simulink model "ofdm_txrx_mimo_2x"  (Simulink 6.6)
settings: Solver=VariableStepDiscrete  StartTime=0.0  StopTime=20e3  RelTol=1e-3
PreLoadFcn:
  | ofdm_rx_mimo_init
InitFcn:
  | ofdm_rx_mimo_init
StartFcn:
  | ofdm_rx_mimo_init
values from neighbouring files (symbols this model uses):
  BER_RAM_init_size = 2048    [ofdm_tx_mimo_init.m]
  CPLength = 16    [ofdm_tx_mimo_init.m]
  CRCPolynomial = hex2dec('04c11db7')    [ofdm_tx_mimo_init.m]
  freqOffset_track_filtCoef_i = hex2dec('2500')/2^32    [ofdm_rx_mimo_init.m]
  freqOffset_track_filtCoef_p = hex2dec('d0000')/2^32    [ofdm_rx_mimo_init.m]
  hi = zeros(1,length(cci))    [ofdm_rx_mimo_init.m]
  hr = zeros(1,length(ccr))    [ofdm_rx_mimo_init.m]
  max_CP_length = 16    [ofdm_tx_mimo_init.m]
  max_num_baseRateSymbols = 31    [ofdm_tx_mimo_init.m]
  max_num_subcarriers = 64    [ofdm_tx_mimo_init.m]
  max_num_trainingSymbols = 15    [ofdm_tx_mimo_init.m]
  max_numBytes = 16384    [ofdm_tx_mimo_init.m]
  max_OFDM_symbols = 2047    [ofdm_tx_mimo_init.m]
  modConstellation_qam16 = .75*[1 3 -1 -3]./3    [ofdm_tx_mimo_init.m]
  modConstellation_qam256 = 0.9375*[3 1 5 7 11 9 13 15 -3 -1 -5 -7 -11 -9 -13 -15]./15    [ofdm_tx_mimo_init.m]
  modConstellation_qam64 = 0.875*[3 1 5 7 -3 -1 -5 -7]./7    [ofdm_tx_mimo_init.m]
  modConstellation_qpsk = [1 -1]./sqrt(2)    [ofdm_tx_mimo_init.m]
  numBaseRateSymbols = 2    [ofdm_tx_mimo_init.m]
  numSubcarriers = 64    [ofdm_tx_mimo_init.m]
  numTrainingSymbols = 2    [ofdm_tx_mimo_init.m]
  pilot1_indicies = 7 + ( (64-7) * 2^16)    (= 3735559)    [ofdm_tx_mimo_init.m]
  pilot2_indicies = 21 + ( (64-21) * 2^16)    (= 2818069)    [ofdm_tx_mimo_init.m]
  pilotValue_neg = hex2dec('8000') + (2^16 * 0)    [ofdm_tx_mimo_init.m]
  pilotValue_pos = hex2dec('7FFF') + (2^16 * 0)    [ofdm_tx_mimo_init.m]
  pkt_crossCorr_thresh = 0.7    [ofdm_rx_mimo_init.m]
  pkt_energy_thresh = 0    [ofdm_rx_mimo_init.m]
  pkt_numPayloadBytes = 1400+42-6    (= 1436)    [ofdm_tx_mimo_init.m]
  pktByteNums = numHeaderBytes +  				byteIndex_numPayloadBytes(1)*2^8 +  				byteIndex_numPayloadBytes(2)*2^16 +  				byteIndex_simpleDynModMasks * 2^24    [ofdm_rx_mimo_init.m]
  PktDet_Delay = 26 + Symbol_Timing_Offset*2^7    (= 1050)    [ofdm_rx_mimo_init.m]
  preamble = 6*[0 shortsyms_10 longsyms_2]    [ofdm_tx_mimo_init.m]
  preamble_I = real(preamble)    [ofdm_tx_mimo_init.m]
  preamble_Q = imag(preamble)    [ofdm_tx_mimo_init.m]
  RAM_init_size = max_numBytes    (= 16384)    [ofdm_tx_mimo_init.m]
  reg_Interrupt_PktBuf_Control = reg_InterruptControl +  	1 * 2^16 +  	0 * 2^24    (= 65656)    [ofdm_rx_mimo_init.m]
  rx_controlBits = 1 * 2  		 + 1 * 4  		 + 0 * 8  		 + 1 * 16 * rx_SISO_Mode  		 + 1 * 32  		 + 1 * 64  		 + 0 * 128  		 + 1 * 256  		 + 0 * 512  		 + 1 * 1024  		 + 0 * 2048  		 + 1 * 4096  		 + 1 * 8192  		 + 0 * 16384  		 + 1 * 65536  		 + 1 * 131072  		 + 0 * 262144  		 + 1 * 524288    [ofdm_rx_mimo_init.m]
  Rx_PhaseNoiseTrack_K = 0.6094    [ofdm_rx_mimo_init.m]
  rx_postEq_scaling = round(rxScaling*2^11) * (1 + 2^16)    [ofdm_rx_mimo_init.m]
  RxDataScrambling_Seq = [40 198 78 63 82 173 102 245 48 111 172 115 147 230 216 93 72 65 62 2 205 242 122 90 128 83 105 97 73 10 5 252]    [ofdm_tx_mimo_init.m]
  simOnly_numSamples = length(preamble)+( (numSubcarriers+CPLength)*(numTrainingSymbols + numBaseRateSymbols + numFullRateSymbols/2) )    [ofdm_tx_mimo_init.m]
  train = [train train]    [ofdm_tx_mimo_init.m]
  tx_controlBits = (antB_preambleShift * 2^4) + (2^0 * tx_SISO_Mode) + 4*0 + 8*1    (= 73)    [ofdm_tx_mimo_init.m]
  TxDataScrambling_Seq = [40 198 78 63 82 173 102 245 48 111 172 115 147 230 216 93 72 65 62 2 205 242 122 90 128 83 105 97 73 10 5 252]    [ofdm_tx_mimo_init.m]
  txReg_symbolCounts = (2^16 * numFullRateSymbols) + (2^8 *numBaseRateSymbols ) + numTrainingSymbols    [ofdm_tx_mimo_init.m]
  TxRx_FFTScaling = bin2dec('010111') + (bin2dec('000101') * 2^6)    [ofdm_tx_mimo_init.m]
undefined symbols (not defined in the file or in its folder): AntA, AntB, BaseRate, CRC_table_gen, gcbh, intfilt, pktTiming_count_payloadStart, pktTiming_symbol_length, xlBlockLoadCallback, xlGetSimulinkPeriod
libraries: xbsIndex_r4 x3973, dspsigops x4, commchan3 x2, commrflib2 x2, dspsrcs4 x1
(part 11 of 38)

== system ofdm_txrx_mimo_2x/OFDM Rx MIMO/Long Corr 4x/C4 ==
  x : Inport
  AddSub : xbsIndex_r4/AddSub  Orientation=down  mode=Addition  use_carryin=off  use_carryout=off  en=off  latency=0  precision=Full  arith_type=Unsigned  n_bits=8  bin_pt=2  quantization=Truncate  overflow=Wrap  dbl_ovrd=off  use_behavioral_HDL=on  pipelined=off  use_rpm=off  hw_selection=Fabric  block_type=addsub
      settings: xl_area=[3 0 0 4 0 0 0]  block_version=VER_STRING_GOES_HERE
  AddSub{1..7,9..14} (13 alike) : xbsIndex_r4/AddSub  Orientation=down  mode=Addition  use_carryin=off  use_carryout=off  en=off  latency=1  precision=Full  arith_type=Unsigned  n_bits=8  bin_pt=2  quantization=Truncate  overflow=Wrap  dbl_ovrd=off  use_behavioral_HDL=on  pipelined=off  use_rpm=off  hw_selection=Fabric  block_type=addsub
      AddSub1 settings: xl_area=[3 5 0 4 0 0 0]  block_version=VER_STRING_GOES_HERE
      AddSub10 settings: xl_area=[3 5 0 4 0 0 0]  block_version=VER_STRING_GOES_HERE
      AddSub11 settings: xl_area=[3 5 0 4 0 0 0]  block_version=VER_STRING_GOES_HERE
      AddSub12 settings: xl_area=[3 5 0 4 0 0 0]  block_version=VER_STRING_GOES_HERE
      AddSub13 settings: xl_area=[3 6 0 5 0 0 0]  block_version=VER_STRING_GOES_HERE
      AddSub14 settings: xl_area=[3 6 0 5 0 0 0]  block_version=VER_STRING_GOES_HERE
      AddSub2 settings: xl_area=[3 6 0 5 0 0 0]  block_version=VER_STRING_GOES_HERE
      AddSub3 settings: xl_area=[3 5 0 4 0 0 0]  block_version=VER_STRING_GOES_HERE
      AddSub4 settings: xl_area=[4 7 0 6 0 0 0]  block_version=VER_STRING_GOES_HERE
      AddSub5 settings: xl_area=[3 5 0 4 0 0 0]  block_version=VER_STRING_GOES_HERE
      AddSub6 settings: xl_area=[3 6 0 5 0 0 0]  block_version=VER_STRING_GOES_HERE
      AddSub7 settings: xl_area=[3 5 0 4 0 0 0]  block_version=VER_STRING_GOES_HERE
      AddSub9 settings: xl_area=[4 7 0 6 0 0 0]  block_version=VER_STRING_GOES_HERE
  AddSub8 : xbsIndex_r4/AddSub  mode=Addition  use_carryin=off  use_carryout=off  en=off  latency=1  precision=Full  arith_type=Unsigned  n_bits=8  bin_pt=2  quantization=Truncate  overflow=Wrap  dbl_ovrd=off  use_behavioral_HDL=on  pipelined=off  use_rpm=off  hw_selection=Fabric  block_type=addsub
      settings: xl_area=[4 8 0 7 0 0 0]  block_version=VER_STRING_GOES_HERE
  C1 : Subsystem  mask{coef2=h(1:N)}
      mask prompts: coef2 "Coefficients"
  C10 : Subsystem  mask{coef2=h(9*N+1:10*N)}
      mask prompts: coef2 "Coefficients"
  C11 : Subsystem  mask{coef2=h(10*N+1:11*N)}
      mask prompts: coef2 "Coefficients"
  C12 : Subsystem  mask{coef2=h(11*N+1:12*N)}
      mask prompts: coef2 "Coefficients"
  C13 : Subsystem  mask{coef2=h(12*N+1:13*N)}
      mask prompts: coef2 "Coefficients"
  C14 : Subsystem  mask{coef2=h(13*N+1:14*N)}
      mask prompts: coef2 "Coefficients"
  C15 : Subsystem  mask{coef2=h(14*N+1:15*N)}
      mask prompts: coef2 "Coefficients"
  C16 : Subsystem  mask{coef2=h(15*N+1:16*N)}
      mask prompts: coef2 "Coefficients"
  C2 : Subsystem  mask{coef2=h(N+1:2*N)}
      mask prompts: coef2 "Coefficients"
  C3 : Subsystem  mask{coef2=h(2*N+1:3*N)}
      mask prompts: coef2 "Coefficients"
  C4 : Subsystem  mask{coef2=h(3*N+1:4*N)}
      mask prompts: coef2 "Coefficients"
  C5 : Subsystem  mask{coef2=h(4*N+1:5*N)}
      mask prompts: coef2 "Coefficients"
  C6 : Subsystem  mask{coef2=h(5*N+1:6*N)}
      mask prompts: coef2 "Coefficients"
  C7 : Subsystem  mask{coef2=h(6*N+1:7*N)}
      mask prompts: coef2 "Coefficients"
  C8 : Subsystem  mask{coef2=h(7*N+1:8*N)}
      mask prompts: coef2 "Coefficients"
  C9 : Subsystem  mask{coef2=h(8*N+1:9*N)}
      mask prompts: coef2 "Coefficients"
  Delay{1,3..4,6,8,12..13,15} (8 alike) : xbsIndex_r4/Delay  Orientation=down  en=off  latency=N-1  dbl_ovrd=off  reg_retiming=off  block_type=delay
      settings: infoedit=Hardware notes: A delay line is a chain, each link of which is an SRL16 followed by a flip-flop. If register retiming is enabled, the delay line is a chain of flip-flops.  xl_area=[2 4 0 4 0 0 0]  block_version=VER_STRING_GOES_HERE
  Delay10 : xbsIndex_r4/Delay  Orientation=down  en=off  latency=((N-1)*12)-1  dbl_ovrd=off  reg_retiming=off  block_type=delay
      settings: infoedit=Hardware notes: A delay line is a chain, each link of which is an SRL16 followed by a flip-flop. If register retiming is enabled, the delay line is a chain of flip-flops.  xl_area=[9 15 0 15 0 0 0]  block_version=VER_STRING_GOES_HERE
  Delay11 : xbsIndex_r4/Delay  Orientation=down  en=off  latency=((N-1)*14)-1  dbl_ovrd=off  reg_retiming=off  block_type=delay
      settings: infoedit=Hardware notes: A delay line is a chain, each link of which is an SRL16 followed by a flip-flop. If register retiming is enabled, the delay line is a chain of flip-flops.  xl_area=[9 15 0 15 0 0 0]  block_version=VER_STRING_GOES_HERE
  Delay14 : xbsIndex_r4/Delay  Orientation=down  en=off  latency=((N-1)*2)-1  dbl_ovrd=off  reg_retiming=off  block_type=delay
      settings: infoedit=Hardware notes: A delay line is a chain, each link of which is an SRL16 followed by a flip-flop. If register retiming is enabled, the delay line is a chain of flip-flops.  xl_area=[3 5 0 5 0 0 0]  block_version=VER_STRING_GOES_HERE
  Delay2 : xbsIndex_r4/Delay  Orientation=down  en=off  latency=((N-1)*4)-1  dbl_ovrd=off  reg_retiming=off  block_type=delay
      settings: infoedit=Hardware notes: A delay line is a chain, each link of which is an SRL16 followed by a flip-flop. If register retiming is enabled, the delay line is a chain of flip-flops.  xl_area=[3 5 0 5 0 0 0]  block_version=VER_STRING_GOES_HERE
  Delay5 : xbsIndex_r4/Delay  Orientation=down  en=off  latency=((N-1)*6)-1  dbl_ovrd=off  reg_retiming=off  block_type=delay
      settings: infoedit=Hardware notes: A delay line is a chain, each link of which is an SRL16 followed by a flip-flop. If register retiming is enabled, the delay line is a chain of flip-flops.  xl_area=[6 10 0 10 0 0 0]  block_version=VER_STRING_GOES_HERE
  Delay7 : xbsIndex_r4/Delay  Orientation=down  en=off  latency=((N-1)*8)-1  dbl_ovrd=off  reg_retiming=off  block_type=delay
      settings: infoedit=Hardware notes: A delay line is a chain, each link of which is an SRL16 followed by a flip-flop. If register retiming is enabled, the delay line is a chain of flip-flops.  xl_area=[6 10 0 10 0 0 0]  block_version=VER_STRING_GOES_HERE
  Delay9 : xbsIndex_r4/Delay  Orientation=down  en=off  latency=((N-1)*10)-1  dbl_ovrd=off  reg_retiming=off  block_type=delay
      settings: infoedit=Hardware notes: A delay line is a chain, each link of which is an SRL16 followed by a flip-flop. If register retiming is enabled, the delay line is a chain of flip-flops.  xl_area=[9 15 0 15 0 0 0]  block_version=VER_STRING_GOES_HERE
  FSM : Subsystem
  Gateway Out6 : xbsIndex_r4/Gateway Out  hdl_port=off  timing_constraint=None  locs_specified=off  LOCs={}  block_type=gatewayout
      settings: infoedit="Gateway out block.  Converts Xilinx fixed point inputs into ouputs of type Simulink integer, double, or fixed point.<P><P>Hardware notes:  In hardware these blocks become top level output ports or are discarded, depending on how they are configured."  xl_area=[0 0 0 0 0 0 0]  block_version=VER_STRING_GOES_HERE
  Gateway Out7 : xbsIndex_r4/Gateway Out  hdl_port=off  timing_constraint=None  locs_specified=off  LOCs={}  block_type=gatewayout
      settings: infoedit="Gateway out block.  Converts Xilinx fixed point inputs into ouputs of type Simulink integer, double, or fixed point.<P><P>Hardware notes:  In hardware these blocks become top level output ports or are discarded, depending on how they are configured."  xl_area=[0 0 0 0 0 0 0]  block_version=VER_STRING_GOES_HERE
  Gateway Out8 : xbsIndex_r4/Gateway Out  hdl_port=off  timing_constraint=None  locs_specified=off  LOCs={}  block_type=gatewayout
      settings: infoedit="Gateway out block.  Converts Xilinx fixed point inputs into ouputs of type Simulink integer, double, or fixed point.<P><P>Hardware notes:  In hardware these blocks become top level output ports or are discarded, depending on how they are configured."  xl_area=[0 0 0 0 0 0 0]  block_version=VER_STRING_GOES_HERE
  Long Corr Scope2 : Scope  NumInputPorts=3
      settings: YMin=-0.35~-0.175~0  YMax=0.35~0.225~1300  SaveName=ScopeData8
  y : Outport
  wires:
  AddSub9/1 -> Gateway Out8/1, AddSub8/2
  AddSub6/1 -> AddSub9/1, Gateway Out7/1
  C13/1(yn) -> AddSub10/1
  AddSub8/1 -> y/1
  Delay9/1 -> AddSub6/2
  Delay7/1 -> AddSub6/1
  C12/1(yn) -> Delay8/1
  AddSub7/1 -> Delay9/1
  Delay8/1 -> AddSub7/2
  C11/1(yn) -> AddSub7/1
  AddSub4/1 -> AddSub8/1
  AddSub2/1 -> AddSub4/2
  AddSub13/1 -> AddSub4/1
  Delay5/1 -> AddSub2/2
  Delay2/1 -> AddSub2/1
  AddSub/1 -> AddSub13/1
  Delay14/1 -> AddSub13/2
  C8/2(xnz) -> [xnz]
  C10/1(yn) -> Delay6/1
  AddSub5/1 -> Delay7/1
  Delay6/1 -> AddSub5/2
  C9/1(yn) -> AddSub5/1
  C8/1(yn) -> Delay4/1
  C7/1(yn) -> AddSub3/1
  AddSub3/1 -> Delay5/1
  Delay4/1 -> AddSub3/2
  C7/2(xnz) -> C8/1(xn)
  C6/1(yn) -> Delay1/1
  AddSub1/1 -> Delay2/1
  Delay1/1 -> AddSub1/2
  C5/1(yn) -> AddSub1/1
  C1/1(yn) -> AddSub/1
  Delay3/1 -> AddSub/2
  AddSub12/1 -> Delay14/1
  Delay13/1 -> AddSub12/2
  C12/2(xnz) -> C13/1(xn)
  C11/2(xnz) -> C12/1(xn)
  C10/2(xnz) -> C11/1(xn)
  C9/2(xnz) -> C10/1(xn)
  [xnz] -> C9/1(xn)
  C6/2(xnz) -> C7/1(xn)
  C5/2(xnz) -> C6/1(xn)
  C4/1(yn) -> Delay13/1
  C4/2(xnz) -> C5/1(xn)
  C3/1(yn) -> AddSub12/1
  C3/2(xnz) -> C4/1(xn)
  C2/2(xnz) -> C3/1(xn)
  FSM/3(load) -> C12/4(LD), C13/4(LD), C14/4(LD), C16/4(LD), C15/4(LD), C11/4(LD), C10/4(LD), C9/4(LD), C1/4(LD), C8/4(LD), C7/4(LD), C6/4(LD), C5/4(LD), C4/4(LD), C3/4(LD), C2/4(LD)
  FSM/2(Coef Addr) -> C12/3(CAddr), C13/3(CAddr), C14/3(CAddr), C16/3(CAddr), C15/3(CAddr), C11/3(CAddr), C10/3(CAddr), C9/3(CAddr), C1/3(CAddr), C8/3(CAddr), C7/3(CAddr), C6/3(CAddr), C5/3(CAddr), C4/3(CAddr), C3/3(CAddr), C2/3(CAddr)
  FSM/1(Data Addr) -> C12/2(DAddr), C13/2(DAddr), C14/2(DAddr), C16/2(DAddr), C15/2(DAddr), C11/2(DAddr), C10/2(DAddr), C9/2(DAddr), C1/2(DAddr), C8/2(DAddr), C7/2(DAddr), C6/2(DAddr), C5/2(DAddr), C4/2(DAddr), C3/2(DAddr), C2/2(DAddr)
  C1/2(xnz) -> C2/1(xn)
  C2/1(yn) -> Delay3/1
  x/1 -> C1/1(xn)
  C13/2(xnz) -> C14/1(xn)
  C14/2(xnz) -> C15/1(xn)
  C15/2(xnz) -> C16/1(xn)
  C15/1(yn) -> AddSub11/1
  C14/1(yn) -> Delay12/1
  C16/1(yn) -> Delay15/1
  Delay12/1 -> AddSub10/2
  Delay15/1 -> AddSub11/2
  AddSub10/1 -> Delay10/1
  AddSub11/1 -> Delay11/1
  Delay10/1 -> AddSub14/1
  Delay11/1 -> AddSub14/2
  AddSub14/1 -> AddSub9/2, Gateway Out6/1
  Gateway Out6/1 -> Long Corr Scope2/1
  Gateway Out7/1 -> Long Corr Scope2/2
  Gateway Out8/1 -> Long Corr Scope2/3

== system ofdm_txrx_mimo_2x/OFDM Rx MIMO/Long Corr 4x/C6 ==
  x : Inport
  AddSub : xbsIndex_r4/AddSub  Orientation=down  mode=Addition  use_carryin=off  use_carryout=off  en=off  latency=0  precision=Full  arith_type=Unsigned  n_bits=8  bin_pt=2  quantization=Truncate  overflow=Wrap  dbl_ovrd=off  use_behavioral_HDL=on  pipelined=off  use_rpm=off  hw_selection=Fabric  block_type=addsub
      settings: xl_area=[3 0 0 4 0 0 0]  block_version=VER_STRING_GOES_HERE
  AddSub{1..7,9..14} (13 alike) : xbsIndex_r4/AddSub  Orientation=down  mode=Addition  use_carryin=off  use_carryout=off  en=off  latency=1  precision=Full  arith_type=Unsigned  n_bits=8  bin_pt=2  quantization=Truncate  overflow=Wrap  dbl_ovrd=off  use_behavioral_HDL=on  pipelined=off  use_rpm=off  hw_selection=Fabric  block_type=addsub
      AddSub1 settings: xl_area=[3 5 0 4 0 0 0]  block_version=VER_STRING_GOES_HERE
      AddSub10 settings: xl_area=[3 5 0 4 0 0 0]  block_version=VER_STRING_GOES_HERE
      AddSub11 settings: xl_area=[3 5 0 4 0 0 0]  block_version=VER_STRING_GOES_HERE
      AddSub12 settings: xl_area=[3 5 0 4 0 0 0]  block_version=VER_STRING_GOES_HERE
      AddSub13 settings: xl_area=[3 6 0 5 0 0 0]  block_version=VER_STRING_GOES_HERE
      AddSub14 settings: xl_area=[3 6 0 5 0 0 0]  block_version=VER_STRING_GOES_HERE
      AddSub2 settings: xl_area=[3 6 0 5 0 0 0]  block_version=VER_STRING_GOES_HERE
      AddSub3 settings: xl_area=[3 5 0 4 0 0 0]  block_version=VER_STRING_GOES_HERE
      AddSub4 settings: xl_area=[4 7 0 6 0 0 0]  block_version=VER_STRING_GOES_HERE
      AddSub5 settings: xl_area=[3 5 0 4 0 0 0]  block_version=VER_STRING_GOES_HERE
      AddSub6 settings: xl_area=[3 6 0 5 0 0 0]  block_version=VER_STRING_GOES_HERE
      AddSub7 settings: xl_area=[3 5 0 4 0 0 0]  block_version=VER_STRING_GOES_HERE
      AddSub9 settings: xl_area=[4 7 0 6 0 0 0]  block_version=VER_STRING_GOES_HERE
  AddSub8 : xbsIndex_r4/AddSub  mode=Addition  use_carryin=off  use_carryout=off  en=off  latency=1  precision=Full  arith_type=Unsigned  n_bits=8  bin_pt=2  quantization=Truncate  overflow=Wrap  dbl_ovrd=off  use_behavioral_HDL=on  pipelined=off  use_rpm=off  hw_selection=Fabric  block_type=addsub
      settings: xl_area=[4 8 0 7 0 0 0]  block_version=VER_STRING_GOES_HERE
  C1 : Subsystem  mask{coef2=h(1:N)}
      mask prompts: coef2 "Coefficients"
  C10 : Subsystem  mask{coef2=h(9*N+1:10*N)}
      mask prompts: coef2 "Coefficients"
  C11 : Subsystem  mask{coef2=h(10*N+1:11*N)}
      mask prompts: coef2 "Coefficients"
  C12 : Subsystem  mask{coef2=h(11*N+1:12*N)}
      mask prompts: coef2 "Coefficients"
  C13 : Subsystem  mask{coef2=h(12*N+1:13*N)}
      mask prompts: coef2 "Coefficients"
  C14 : Subsystem  mask{coef2=h(13*N+1:14*N)}
      mask prompts: coef2 "Coefficients"
  C15 : Subsystem  mask{coef2=h(14*N+1:15*N)}
      mask prompts: coef2 "Coefficients"
  C16 : Subsystem  mask{coef2=h(15*N+1:16*N)}
      mask prompts: coef2 "Coefficients"
  C2 : Subsystem  mask{coef2=h(N+1:2*N)}
      mask prompts: coef2 "Coefficients"
  C3 : Subsystem  mask{coef2=h(2*N+1:3*N)}
      mask prompts: coef2 "Coefficients"
  C4 : Subsystem  mask{coef2=h(3*N+1:4*N)}
      mask prompts: coef2 "Coefficients"
  C5 : Subsystem  mask{coef2=h(4*N+1:5*N)}
      mask prompts: coef2 "Coefficients"
  C6 : Subsystem  mask{coef2=h(5*N+1:6*N)}
      mask prompts: coef2 "Coefficients"
  C7 : Subsystem  mask{coef2=h(6*N+1:7*N)}
      mask prompts: coef2 "Coefficients"
  C8 : Subsystem  mask{coef2=h(7*N+1:8*N)}
      mask prompts: coef2 "Coefficients"
  C9 : Subsystem  mask{coef2=h(8*N+1:9*N)}
      mask prompts: coef2 "Coefficients"
  Delay{1,3..4,6,8,12..13,15} (8 alike) : xbsIndex_r4/Delay  Orientation=down  en=off  latency=N-1  dbl_ovrd=off  reg_retiming=off  block_type=delay
      settings: infoedit=Hardware notes: A delay line is a chain, each link of which is an SRL16 followed by a flip-flop. If register retiming is enabled, the delay line is a chain of flip-flops.  xl_area=[2 4 0 4 0 0 0]  block_version=VER_STRING_GOES_HERE
  Delay10 : xbsIndex_r4/Delay  Orientation=down  en=off  latency=((N-1)*12)-1  dbl_ovrd=off  reg_retiming=off  block_type=delay
      settings: infoedit=Hardware notes: A delay line is a chain, each link of which is an SRL16 followed by a flip-flop. If register retiming is enabled, the delay line is a chain of flip-flops.  xl_area=[9 15 0 15 0 0 0]  block_version=VER_STRING_GOES_HERE
  Delay11 : xbsIndex_r4/Delay  Orientation=down  en=off  latency=((N-1)*14)-1  dbl_ovrd=off  reg_retiming=off  block_type=delay
      settings: infoedit=Hardware notes: A delay line is a chain, each link of which is an SRL16 followed by a flip-flop. If register retiming is enabled, the delay line is a chain of flip-flops.  xl_area=[9 15 0 15 0 0 0]  block_version=VER_STRING_GOES_HERE
  Delay14 : xbsIndex_r4/Delay  Orientation=down  en=off  latency=((N-1)*2)-1  dbl_ovrd=off  reg_retiming=off  block_type=delay
      settings: infoedit=Hardware notes: A delay line is a chain, each link of which is an SRL16 followed by a flip-flop. If register retiming is enabled, the delay line is a chain of flip-flops.  xl_area=[3 5 0 5 0 0 0]  block_version=VER_STRING_GOES_HERE
  Delay2 : xbsIndex_r4/Delay  Orientation=down  en=off  latency=((N-1)*4)-1  dbl_ovrd=off  reg_retiming=off  block_type=delay
      settings: infoedit=Hardware notes: A delay line is a chain, each link of which is an SRL16 followed by a flip-flop. If register retiming is enabled, the delay line is a chain of flip-flops.  xl_area=[3 5 0 5 0 0 0]  block_version=VER_STRING_GOES_HERE
  Delay5 : xbsIndex_r4/Delay  Orientation=down  en=off  latency=((N-1)*6)-1  dbl_ovrd=off  reg_retiming=off  block_type=delay
      settings: infoedit=Hardware notes: A delay line is a chain, each link of which is an SRL16 followed by a flip-flop. If register retiming is enabled, the delay line is a chain of flip-flops.  xl_area=[6 10 0 10 0 0 0]  block_version=VER_STRING_GOES_HERE
  Delay7 : xbsIndex_r4/Delay  Orientation=down  en=off  latency=((N-1)*8)-1  dbl_ovrd=off  reg_retiming=off  block_type=delay
      settings: infoedit=Hardware notes: A delay line is a chain, each link of which is an SRL16 followed by a flip-flop. If register retiming is enabled, the delay line is a chain of flip-flops.  xl_area=[6 10 0 10 0 0 0]  block_version=VER_STRING_GOES_HERE
  Delay9 : xbsIndex_r4/Delay  Orientation=down  en=off  latency=((N-1)*10)-1  dbl_ovrd=off  reg_retiming=off  block_type=delay
      settings: infoedit=Hardware notes: A delay line is a chain, each link of which is an SRL16 followed by a flip-flop. If register retiming is enabled, the delay line is a chain of flip-flops.  xl_area=[9 15 0 15 0 0 0]  block_version=VER_STRING_GOES_HERE
  FSM : Subsystem
  Gateway Out6 : xbsIndex_r4/Gateway Out  hdl_port=off  timing_constraint=None  locs_specified=off  LOCs={}  block_type=gatewayout
      settings: infoedit="Gateway out block.  Converts Xilinx fixed point inputs into ouputs of type Simulink integer, double, or fixed point.<P><P>Hardware notes:  In hardware these blocks become top level output ports or are discarded, depending on how they are configured."  xl_area=[0 0 0 0 0 0 0]  block_version=VER_STRING_GOES_HERE
  Gateway Out7 : xbsIndex_r4/Gateway Out  hdl_port=off  timing_constraint=None  locs_specified=off  LOCs={}  block_type=gatewayout
      settings: infoedit="Gateway out block.  Converts Xilinx fixed point inputs into ouputs of type Simulink integer, double, or fixed point.<P><P>Hardware notes:  In hardware these blocks become top level output ports or are discarded, depending on how they are configured."  xl_area=[0 0 0 0 0 0 0]  block_version=VER_STRING_GOES_HERE
  Gateway Out8 : xbsIndex_r4/Gateway Out  hdl_port=off  timing_constraint=None  locs_specified=off  LOCs={}  block_type=gatewayout
      settings: infoedit="Gateway out block.  Converts Xilinx fixed point inputs into ouputs of type Simulink integer, double, or fixed point.<P><P>Hardware notes:  In hardware these blocks become top level output ports or are discarded, depending on how they are configured."  xl_area=[0 0 0 0 0 0 0]  block_version=VER_STRING_GOES_HERE
  Long Corr Scope2 : Scope  NumInputPorts=3
      settings: YMin=-0.35~-0.175~0  YMax=0.35~0.225~1300  SaveName=ScopeData8
  y : Outport
  wires:
  Gateway Out8/1 -> Long Corr Scope2/3
  Gateway Out7/1 -> Long Corr Scope2/2
  Gateway Out6/1 -> Long Corr Scope2/1
  AddSub14/1 -> Gateway Out6/1, AddSub9/2
  Delay11/1 -> AddSub14/2
  Delay10/1 -> AddSub14/1
  AddSub11/1 -> Delay11/1
  AddSub10/1 -> Delay10/1
  Delay15/1 -> AddSub11/2
  Delay12/1 -> AddSub10/2
  C16/1(yn) -> Delay15/1
  C14/1(yn) -> Delay12/1
  C15/1(yn) -> AddSub11/1
  C15/2(xnz) -> C16/1(xn)
  C14/2(xnz) -> C15/1(xn)
  C13/2(xnz) -> C14/1(xn)
  x/1 -> C1/1(xn)
  C2/1(yn) -> Delay3/1
  C1/2(xnz) -> C2/1(xn)
  FSM/1(Data Addr) -> C2/2(DAddr), C3/2(DAddr), C4/2(DAddr), C5/2(DAddr), C6/2(DAddr), C7/2(DAddr), C8/2(DAddr), C1/2(DAddr), C9/2(DAddr), C10/2(DAddr), C11/2(DAddr), C15/2(DAddr), C16/2(DAddr), C14/2(DAddr), C13/2(DAddr), C12/2(DAddr)
  FSM/2(Coef Addr) -> C2/3(CAddr), C3/3(CAddr), C4/3(CAddr), C5/3(CAddr), C6/3(CAddr), C7/3(CAddr), C8/3(CAddr), C1/3(CAddr), C9/3(CAddr), C10/3(CAddr), C11/3(CAddr), C15/3(CAddr), C16/3(CAddr), C14/3(CAddr), C13/3(CAddr), C12/3(CAddr)
  FSM/3(load) -> C2/4(LD), C3/4(LD), C4/4(LD), C5/4(LD), C6/4(LD), C7/4(LD), C8/4(LD), C1/4(LD), C9/4(LD), C10/4(LD), C11/4(LD), C15/4(LD), C16/4(LD), C14/4(LD), C13/4(LD), C12/4(LD)
  C2/2(xnz) -> C3/1(xn)
  C3/2(xnz) -> C4/1(xn)
  C3/1(yn) -> AddSub12/1
  C4/2(xnz) -> C5/1(xn)
  C4/1(yn) -> Delay13/1
  C5/2(xnz) -> C6/1(xn)
  C6/2(xnz) -> C7/1(xn)
  [xnz] -> C9/1(xn)
  C9/2(xnz) -> C10/1(xn)
  C10/2(xnz) -> C11/1(xn)
  C11/2(xnz) -> C12/1(xn)
  C12/2(xnz) -> C13/1(xn)
  Delay13/1 -> AddSub12/2
  AddSub12/1 -> Delay14/1
  Delay3/1 -> AddSub/2
  C1/1(yn) -> AddSub/1
  C5/1(yn) -> AddSub1/1
  Delay1/1 -> AddSub1/2
  AddSub1/1 -> Delay2/1
  C6/1(yn) -> Delay1/1
  C7/2(xnz) -> C8/1(xn)
  Delay4/1 -> AddSub3/2
  AddSub3/1 -> Delay5/1
  C7/1(yn) -> AddSub3/1
  C8/1(yn) -> Delay4/1
  C9/1(yn) -> AddSub5/1
  Delay6/1 -> AddSub5/2
  AddSub5/1 -> Delay7/1
  C10/1(yn) -> Delay6/1
  C8/2(xnz) -> [xnz]
  Delay14/1 -> AddSub13/2
  AddSub/1 -> AddSub13/1
  Delay2/1 -> AddSub2/1
  Delay5/1 -> AddSub2/2
  AddSub13/1 -> AddSub4/1
  AddSub2/1 -> AddSub4/2
  AddSub4/1 -> AddSub8/1
  C11/1(yn) -> AddSub7/1
  Delay8/1 -> AddSub7/2
  AddSub7/1 -> Delay9/1
  C12/1(yn) -> Delay8/1
  Delay7/1 -> AddSub6/1
  Delay9/1 -> AddSub6/2
  AddSub8/1 -> y/1
  C13/1(yn) -> AddSub10/1
  AddSub6/1 -> Gateway Out7/1, AddSub9/1
  AddSub9/1 -> AddSub8/2, Gateway Out8/1

== system ofdm_txrx_mimo_2x/OFDM Rx MIMO/Long Corr 4x/C7 ==
  x : Inport
  AddSub : xbsIndex_r4/AddSub  Orientation=down  mode=Addition  use_carryin=off  use_carryout=off  en=off  latency=0  precision=Full  arith_type=Unsigned  n_bits=8  bin_pt=2  quantization=Truncate  overflow=Wrap  dbl_ovrd=off  use_behavioral_HDL=on  pipelined=off  use_rpm=off  hw_selection=Fabric  block_type=addsub
      settings: xl_area=[3 0 0 4 0 0 0]  block_version=VER_STRING_GOES_HERE
  AddSub{1..7,9..14} (13 alike) : xbsIndex_r4/AddSub  Orientation=down  mode=Addition  use_carryin=off  use_carryout=off  en=off  latency=1  precision=Full  arith_type=Unsigned  n_bits=8  bin_pt=2  quantization=Truncate  overflow=Wrap  dbl_ovrd=off  use_behavioral_HDL=on  pipelined=off  use_rpm=off  hw_selection=Fabric  block_type=addsub
      AddSub1 settings: xl_area=[3 5 0 4 0 0 0]  block_version=VER_STRING_GOES_HERE
      AddSub10 settings: xl_area=[3 5 0 4 0 0 0]  block_version=VER_STRING_GOES_HERE
      AddSub11 settings: xl_area=[3 5 0 4 0 0 0]  block_version=VER_STRING_GOES_HERE
      AddSub12 settings: xl_area=[3 5 0 4 0 0 0]  block_version=VER_STRING_GOES_HERE
      AddSub13 settings: xl_area=[3 6 0 5 0 0 0]  block_version=VER_STRING_GOES_HERE
      AddSub14 settings: xl_area=[3 6 0 5 0 0 0]  block_version=VER_STRING_GOES_HERE
      AddSub2 settings: xl_area=[3 6 0 5 0 0 0]  block_version=VER_STRING_GOES_HERE
      AddSub3 settings: xl_area=[3 5 0 4 0 0 0]  block_version=VER_STRING_GOES_HERE
      AddSub4 settings: xl_area=[4 7 0 6 0 0 0]  block_version=VER_STRING_GOES_HERE
      AddSub5 settings: xl_area=[3 5 0 4 0 0 0]  block_version=VER_STRING_GOES_HERE
      AddSub6 settings: xl_area=[3 6 0 5 0 0 0]  block_version=VER_STRING_GOES_HERE
      AddSub7 settings: xl_area=[3 5 0 4 0 0 0]  block_version=VER_STRING_GOES_HERE
      AddSub9 settings: xl_area=[4 7 0 6 0 0 0]  block_version=VER_STRING_GOES_HERE
  AddSub8 : xbsIndex_r4/AddSub  mode=Addition  use_carryin=off  use_carryout=off  en=off  latency=1  precision=Full  arith_type=Unsigned  n_bits=8  bin_pt=2  quantization=Truncate  overflow=Wrap  dbl_ovrd=off  use_behavioral_HDL=on  pipelined=off  use_rpm=off  hw_selection=Fabric  block_type=addsub
      settings: xl_area=[4 8 0 7 0 0 0]  block_version=VER_STRING_GOES_HERE
  C1 : Subsystem  mask{coef2=h(1:N)}
      mask prompts: coef2 "Coefficients"
  C10 : Subsystem  mask{coef2=h(9*N+1:10*N)}
      mask prompts: coef2 "Coefficients"
  C11 : Subsystem  mask{coef2=h(10*N+1:11*N)}
      mask prompts: coef2 "Coefficients"
  C12 : Subsystem  mask{coef2=h(11*N+1:12*N)}
      mask prompts: coef2 "Coefficients"
  C13 : Subsystem  mask{coef2=h(12*N+1:13*N)}
      mask prompts: coef2 "Coefficients"
  C14 : Subsystem  mask{coef2=h(13*N+1:14*N)}
      mask prompts: coef2 "Coefficients"
  C15 : Subsystem  mask{coef2=h(14*N+1:15*N)}
      mask prompts: coef2 "Coefficients"
  C16 : Subsystem  mask{coef2=h(15*N+1:16*N)}
      mask prompts: coef2 "Coefficients"
  C2 : Subsystem  mask{coef2=h(N+1:2*N)}
      mask prompts: coef2 "Coefficients"
  C3 : Subsystem  mask{coef2=h(2*N+1:3*N)}
      mask prompts: coef2 "Coefficients"
  C4 : Subsystem  mask{coef2=h(3*N+1:4*N)}
      mask prompts: coef2 "Coefficients"
  C5 : Subsystem  mask{coef2=h(4*N+1:5*N)}
      mask prompts: coef2 "Coefficients"
  C6 : Subsystem  mask{coef2=h(5*N+1:6*N)}
      mask prompts: coef2 "Coefficients"
  C7 : Subsystem  mask{coef2=h(6*N+1:7*N)}
      mask prompts: coef2 "Coefficients"
  C8 : Subsystem  mask{coef2=h(7*N+1:8*N)}
      mask prompts: coef2 "Coefficients"
  C9 : Subsystem  mask{coef2=h(8*N+1:9*N)}
      mask prompts: coef2 "Coefficients"
  Delay{1,3..4,6,8,12..13,15} (8 alike) : xbsIndex_r4/Delay  Orientation=down  en=off  latency=N-1  dbl_ovrd=off  reg_retiming=off  block_type=delay
      settings: infoedit=Hardware notes: A delay line is a chain, each link of which is an SRL16 followed by a flip-flop. If register retiming is enabled, the delay line is a chain of flip-flops.  xl_area=[2 4 0 4 0 0 0]  block_version=VER_STRING_GOES_HERE
  Delay10 : xbsIndex_r4/Delay  Orientation=down  en=off  latency=((N-1)*12)-1  dbl_ovrd=off  reg_retiming=off  block_type=delay
      settings: infoedit=Hardware notes: A delay line is a chain, each link of which is an SRL16 followed by a flip-flop. If register retiming is enabled, the delay line is a chain of flip-flops.  xl_area=[9 15 0 15 0 0 0]  block_version=VER_STRING_GOES_HERE
  Delay11 : xbsIndex_r4/Delay  Orientation=down  en=off  latency=((N-1)*14)-1  dbl_ovrd=off  reg_retiming=off  block_type=delay
      settings: infoedit=Hardware notes: A delay line is a chain, each link of which is an SRL16 followed by a flip-flop. If register retiming is enabled, the delay line is a chain of flip-flops.  xl_area=[9 15 0 15 0 0 0]  block_version=VER_STRING_GOES_HERE
  Delay14 : xbsIndex_r4/Delay  Orientation=down  en=off  latency=((N-1)*2)-1  dbl_ovrd=off  reg_retiming=off  block_type=delay
      settings: infoedit=Hardware notes: A delay line is a chain, each link of which is an SRL16 followed by a flip-flop. If register retiming is enabled, the delay line is a chain of flip-flops.  xl_area=[3 5 0 5 0 0 0]  block_version=VER_STRING_GOES_HERE
  Delay2 : xbsIndex_r4/Delay  Orientation=down  en=off  latency=((N-1)*4)-1  dbl_ovrd=off  reg_retiming=off  block_type=delay
      settings: infoedit=Hardware notes: A delay line is a chain, each link of which is an SRL16 followed by a flip-flop. If register retiming is enabled, the delay line is a chain of flip-flops.  xl_area=[3 5 0 5 0 0 0]  block_version=VER_STRING_GOES_HERE
  Delay5 : xbsIndex_r4/Delay  Orientation=down  en=off  latency=((N-1)*6)-1  dbl_ovrd=off  reg_retiming=off  block_type=delay
      settings: infoedit=Hardware notes: A delay line is a chain, each link of which is an SRL16 followed by a flip-flop. If register retiming is enabled, the delay line is a chain of flip-flops.  xl_area=[6 10 0 10 0 0 0]  block_version=VER_STRING_GOES_HERE
  Delay7 : xbsIndex_r4/Delay  Orientation=down  en=off  latency=((N-1)*8)-1  dbl_ovrd=off  reg_retiming=off  block_type=delay
      settings: infoedit=Hardware notes: A delay line is a chain, each link of which is an SRL16 followed by a flip-flop. If register retiming is enabled, the delay line is a chain of flip-flops.  xl_area=[6 10 0 10 0 0 0]  block_version=VER_STRING_GOES_HERE
  Delay9 : xbsIndex_r4/Delay  Orientation=down  en=off  latency=((N-1)*10)-1  dbl_ovrd=off  reg_retiming=off  block_type=delay
      settings: infoedit=Hardware notes: A delay line is a chain, each link of which is an SRL16 followed by a flip-flop. If register retiming is enabled, the delay line is a chain of flip-flops.  xl_area=[9 15 0 15 0 0 0]  block_version=VER_STRING_GOES_HERE
  FSM : Subsystem
  Gateway Out6 : xbsIndex_r4/Gateway Out  hdl_port=off  timing_constraint=None  locs_specified=off  LOCs={}  block_type=gatewayout
      settings: infoedit="Gateway out block.  Converts Xilinx fixed point inputs into ouputs of type Simulink integer, double, or fixed point.<P><P>Hardware notes:  In hardware these blocks become top level output ports or are discarded, depending on how they are configured."  xl_area=[0 0 0 0 0 0 0]  block_version=VER_STRING_GOES_HERE
  Gateway Out7 : xbsIndex_r4/Gateway Out  hdl_port=off  timing_constraint=None  locs_specified=off  LOCs={}  block_type=gatewayout
      settings: infoedit="Gateway out block.  Converts Xilinx fixed point inputs into ouputs of type Simulink integer, double, or fixed point.<P><P>Hardware notes:  In hardware these blocks become top level output ports or are discarded, depending on how they are configured."  xl_area=[0 0 0 0 0 0 0]  block_version=VER_STRING_GOES_HERE
  Gateway Out8 : xbsIndex_r4/Gateway Out  hdl_port=off  timing_constraint=None  locs_specified=off  LOCs={}  block_type=gatewayout
      settings: infoedit="Gateway out block.  Converts Xilinx fixed point inputs into ouputs of type Simulink integer, double, or fixed point.<P><P>Hardware notes:  In hardware these blocks become top level output ports or are discarded, depending on how they are configured."  xl_area=[0 0 0 0 0 0 0]  block_version=VER_STRING_GOES_HERE
  Long Corr Scope2 : Scope  NumInputPorts=3
      settings: YMin=-0.35~-0.175~0  YMax=0.35~0.225~1300  SaveName=ScopeData8
  y : Outport
  wires:
  AddSub9/1 -> Gateway Out8/1, AddSub8/2
  AddSub6/1 -> AddSub9/1, Gateway Out7/1
  C13/1(yn) -> AddSub10/1
  AddSub8/1 -> y/1
  Delay9/1 -> AddSub6/2
  Delay7/1 -> AddSub6/1
  C12/1(yn) -> Delay8/1
  AddSub7/1 -> Delay9/1
  Delay8/1 -> AddSub7/2
  C11/1(yn) -> AddSub7/1
  AddSub4/1 -> AddSub8/1
  AddSub2/1 -> AddSub4/2
  AddSub13/1 -> AddSub4/1
  Delay5/1 -> AddSub2/2
  Delay2/1 -> AddSub2/1
  AddSub/1 -> AddSub13/1
  Delay14/1 -> AddSub13/2
  C8/2(xnz) -> [xnz]
  C10/1(yn) -> Delay6/1
  AddSub5/1 -> Delay7/1
  Delay6/1 -> AddSub5/2
  C9/1(yn) -> AddSub5/1
  C8/1(yn) -> Delay4/1
  C7/1(yn) -> AddSub3/1
  AddSub3/1 -> Delay5/1
  Delay4/1 -> AddSub3/2
  C7/2(xnz) -> C8/1(xn)
  C6/1(yn) -> Delay1/1
  AddSub1/1 -> Delay2/1
  Delay1/1 -> AddSub1/2
  C5/1(yn) -> AddSub1/1
  C1/1(yn) -> AddSub/1
  Delay3/1 -> AddSub/2
  AddSub12/1 -> Delay14/1
  Delay13/1 -> AddSub12/2
  C12/2(xnz) -> C13/1(xn)
  C11/2(xnz) -> C12/1(xn)
  C10/2(xnz) -> C11/1(xn)
  C9/2(xnz) -> C10/1(xn)
  [xnz] -> C9/1(xn)
  C6/2(xnz) -> C7/1(xn)
  C5/2(xnz) -> C6/1(xn)
  C4/1(yn) -> Delay13/1
  C4/2(xnz) -> C5/1(xn)
  C3/1(yn) -> AddSub12/1
  C3/2(xnz) -> C4/1(xn)
  C2/2(xnz) -> C3/1(xn)
  FSM/3(load) -> C12/4(LD), C13/4(LD), C14/4(LD), C16/4(LD), C15/4(LD), C11/4(LD), C10/4(LD), C9/4(LD), C1/4(LD), C8/4(LD), C7/4(LD), C6/4(LD), C5/4(LD), C4/4(LD), C3/4(LD), C2/4(LD)
  FSM/2(Coef Addr) -> C12/3(CAddr), C13/3(CAddr), C14/3(CAddr), C16/3(CAddr), C15/3(CAddr), C11/3(CAddr), C10/3(CAddr), C9/3(CAddr), C1/3(CAddr), C8/3(CAddr), C7/3(CAddr), C6/3(CAddr), C5/3(CAddr), C4/3(CAddr), C3/3(CAddr), C2/3(CAddr)
  FSM/1(Data Addr) -> C12/2(DAddr), C13/2(DAddr), C14/2(DAddr), C16/2(DAddr), C15/2(DAddr), C11/2(DAddr), C10/2(DAddr), C9/2(DAddr), C1/2(DAddr), C8/2(DAddr), C7/2(DAddr), C6/2(DAddr), C5/2(DAddr), C4/2(DAddr), C3/2(DAddr), C2/2(DAddr)
  C1/2(xnz) -> C2/1(xn)
  C2/1(yn) -> Delay3/1
  x/1 -> C1/1(xn)
  C13/2(xnz) -> C14/1(xn)
  C14/2(xnz) -> C15/1(xn)
  C15/2(xnz) -> C16/1(xn)
  C15/1(yn) -> AddSub11/1
  C14/1(yn) -> Delay12/1
  C16/1(yn) -> Delay15/1
  Delay12/1 -> AddSub10/2
  Delay15/1 -> AddSub11/2
  AddSub10/1 -> Delay10/1
  AddSub11/1 -> Delay11/1
  Delay10/1 -> AddSub14/1
  Delay11/1 -> AddSub14/2
  AddSub14/1 -> AddSub9/2, Gateway Out6/1
  Gateway Out6/1 -> Long Corr Scope2/1
  Gateway Out7/1 -> Long Corr Scope2/2
  Gateway Out8/1 -> Long Corr Scope2/3

== system ofdm_txrx_mimo_2x/OFDM Rx MIMO/Packet_Detection/A*conj(B) 4xCLK ==
  Re[A] : Inport
  Re[B] : Inport  Port=2
  Im[A] : Inport  Port=3
  Im[B] : Inport  Port=4
  Add1 : xbsIndex_r4/AddSub  mode=Addition  use_carryin=off  use_carryout=off  en=off  latency=0  precision=User Defined  arith_type="Signed  (2's comp)"  n_bits=17  bin_pt=15  quantization=Truncate  overflow=Flag as error  dbl_ovrd=off  use_behavioral_HDL=on  pipelined=off  use_rpm=on  hw_selection=Fabric  block_type=addsub
      settings: xl_area=[9 0 0 16 0 0 0]  block_version=VER_STRING_GOES_HERE
  Counter : xbsIndex_r4/Counter  Orientation=down  cnt_type=Free Running  cnt_to=4  operation=Up  start_count=0  cnt_by_val=1  arith_type=Unsigned  n_bits=2  bin_pt=0  load_pin=off  rst=off  en=off  explicit_period=on  period=1  dbl_ovrd=off  use_behavioral_HDL=off  use_rpm=on  implementation=Fabric  block_type=counter
      settings: xl_area=[3 3 0 4 0 0 0]  block_version=VER_STRING_GOES_HERE
  Delay1 : xbsIndex_r4/Delay  en=off  latency=1  dbl_ovrd=off  reg_retiming=off  block_type=delay
      settings: infoedit=Hardware notes: A delay line is a chain, each link of which is an SRL16 followed by a flip-flop. If register retiming is enabled, the delay line is a chain of flip-flops.  xl_area=[8 16 0 0 0 0 0]  block_version=VER_STRING_GOES_HERE
  Delay2 : xbsIndex_r4/Delay  en=off  latency=2  dbl_ovrd=off  reg_retiming=off  block_type=delay
      settings: infoedit=Hardware notes: A delay line is a chain, each link of which is an SRL16 followed by a flip-flop. If register retiming is enabled, the delay line is a chain of flip-flops.  xl_area=[9 17 0 17 0 0 0]  block_version=VER_STRING_GOES_HERE
  Delay3 : xbsIndex_r4/Delay  en=off  latency=0  dbl_ovrd=off  reg_retiming=off  block_type=delay
      settings: infoedit=Hardware notes: A delay line is a chain, each link of which is an SRL16 followed by a flip-flop. If register retiming is enabled, the delay line is a chain of flip-flops.  xl_area=[9 17 0 17 0 0 0]  block_version=VER_STRING_GOES_HERE
  Down Sample3 : xbsIndex_r4/Down Sample  sample_ratio=4  sample_phase=First Value of Frame  en=off  latency=1  dbl_ovrd=off  block_type=dsamp
      settings: xl_area=[9 17 0 0 0 0 0]  block_version=VER_STRING_GOES_HERE
  Down Sample4 : xbsIndex_r4/Down Sample  sample_ratio=4  sample_phase=First Value of Frame  en=off  latency=1  dbl_ovrd=off  block_type=dsamp
      settings: xl_area=[9 17 0 0 0 0 0]  block_version=VER_STRING_GOES_HERE
  Mult4 : xbsIndex_r4/Mult  precision=User Defined  arith_type="Signed  (2's comp)"  n_bits=16  bin_pt=15  quantization=Truncate  overflow=Flag as error  en=off  latency=1  dbl_ovrd=off  use_behavioral_HDL=off  use_embedded=on  opt=Speed  optimum_pipeline=off  pipeline=on  use_rpm=off  placement_style=Rectangular shape  block_type=mult
      settings: infoedit=Hardware notes: To use the internal pipeline stage of the dedicated multiplier you must select 'Pipeline for maximum performance'.  xl_area=[14 27 0 27 0 1 0]  block_version=VER_STRING_GOES_HERE
  Mux : xbsIndex_r4/Mux  inputs=4  en=off  latency=0  precision=Full  arith_type="Signed  (2's comp)"  n_bits=16  bin_pt=13  quantization=Truncate  overflow=Wrap  dbl_ovrd=off  block_type=mux
      settings: xl_area=[14 0 0 28 0 0 0]  block_version=VER_STRING_GOES_HERE
  Mux1 : xbsIndex_r4/Mux  inputs=4  en=off  latency=0  precision=Full  arith_type="Signed  (2's comp)"  n_bits=16  bin_pt=13  quantization=Truncate  overflow=Wrap  dbl_ovrd=off  block_type=mux
      settings: xl_area=[14 0 0 28 0 0 0]  block_version=VER_STRING_GOES_HERE
  Negate : xbsIndex_r4/Negate  precision=User Defined  arith_type="Signed  (2's comp)"  n_bits=14  bin_pt=13  quantization=Truncate  overflow=Wrap  en=off  latency=0  dbl_ovrd=off  block_type=negate
      settings: xl_area=[7 0 0 14 0 0 0]  block_version=VER_STRING_GOES_HERE
  Negate1 : xbsIndex_r4/Negate  precision=User Defined  arith_type="Signed  (2's comp)"  n_bits=14  bin_pt=13  quantization=Truncate  overflow=Wrap  en=off  latency=0  dbl_ovrd=off  block_type=negate
      settings: xl_area=[7 0 0 14 0 0 0]  block_version=VER_STRING_GOES_HERE
  Slice : xbsIndex_r4/Slice  nbits=2  boolean_output=off  mode=Lower Bit Location + Width  bit1=0  base1=MSB of Input  bit0=0  base0=LSB of Input  dbl_ovrd=off  block_type=slice
      settings: infoedit="Extracts a given range of bits from each input sample and presents it at the output.  The output type is ordinarily unsigned with binary point at zero, but can be Boolean when the slice is one bit wide.<P><P>Hardware notes: In hardware this block costs nothing."  block_version=VER_STRING_GOES_HERE
  Up Sample{1..4} (4 alike) : xbsIndex_r4/Up Sample  sample_ratio=4  copy_samples=on  dbl_ovrd=off  block_type=usamp
      settings: infoedit="Up samples input data.  Inserted values  can be zeros or copies of the most recent input sample.<P><P>Hardware notes: No hardware is needed if inserted values are copies of the input sample; otherwise, a mux and single bit flip-flop are used."  xl_area=[0 0 0 0 0 0 0]  block_version=VER_STRING_GOES_HERE
  Re : Outport
  Im1 : Outport  Port=2
  wires:
  Delay3/1 -> Down Sample4/1
  Slice/1 -> Mux/1, Mux1/1
  Counter/1 -> Slice/1
  Delay1/1 -> Add1/2
  Mult4/1 -> Delay1/1, Add1/1
  Up Sample1/1 -> Mux1/5, Negate/1
  Up Sample4/1 -> Negate1/1
  Up Sample2/1 -> Mux/2, Mux/5
  Up Sample3/1 -> Mux1/4, Mux1/2
  Re[A]/1 -> Up Sample3/1
  Re[B]/1 -> Up Sample2/1
  Im[A]/1 -> Up Sample1/1
  Im[B]/1 -> Up Sample4/1
  Mux1/1 -> Mult4/1
  Mux/1 -> Mult4/2
  Delay2/1 -> Down Sample3/1
  Down Sample4/1 -> Im1/1
  Down Sample3/1 -> Re/1
  Add1/1 -> Delay2/1, Delay3/1
  Negate1/1 -> Mux/4, Mux/3
  Negate/1 -> Mux1/3

== system ofdm_txrx_mimo_2x/OFDM Rx MIMO/Packet_Detection/Magnitude Squared ==
  I : Inport
  Q : Inport  Port=2
  AddSub1 : xbsIndex_r4/AddSub  mode=Addition  use_carryin=off  use_carryout=off  en=off  latency=addLatency  precision=User Defined  arith_type=Unsigned  n_bits=prec  bin_pt=bp  quantization=Truncate  overflow=Flag as error  dbl_ovrd=off  use_behavioral_HDL=on  pipelined=off  use_rpm=on  hw_selection=Fabric  block_type=addsub
      settings: xl_area=[12 22 0 22 0 0 0]  block_version=VER_STRING_GOES_HERE
  Mult1 : xbsIndex_r4/Mult  precision=User Defined  arith_type="Signed  (2's comp)"  n_bits=prec  bin_pt=bp+1  quantization=Truncate  overflow=Flag as error  en=off  latency=multLatency  dbl_ovrd=off  use_behavioral_HDL=off  use_embedded=on  opt=Speed  optimum_pipeline=off  pipeline=on  use_rpm=off  placement_style=Rectangular shape  block_type=mult
      settings: infoedit=Hardware notes: To use the internal pipeline stage of the dedicated multiplier you must select 'Pipeline for maximum performance'.  xl_area=[63 105 0 106 0 4 0]  block_version=VER_STRING_GOES_HERE
  Mult4 : xbsIndex_r4/Mult  precision=User Defined  arith_type="Signed  (2's comp)"  n_bits=prec  bin_pt=bp+1  quantization=Truncate  overflow=Flag as error  en=off  latency=multLatency  dbl_ovrd=off  use_behavioral_HDL=off  use_embedded=on  opt=Speed  optimum_pipeline=off  pipeline=on  use_rpm=off  placement_style=Rectangular shape  block_type=mult
      settings: infoedit=Hardware notes: To use the internal pipeline stage of the dedicated multiplier you must select 'Pipeline for maximum performance'.  xl_area=[63 105 0 106 0 4 0]  block_version=VER_STRING_GOES_HERE
  Mag : Outport
  wires:
  AddSub1/1 -> Mag/1
  Mult1/1 -> AddSub1/2
  Mult4/1 -> AddSub1/1
  Q/1 -> Mult1/2, Mult1/1
  I/1 -> Mult4/2, Mult4/1

== system ofdm_txrx_mimo_2x/OFDM Rx MIMO/Packet_Detection/Magnitude Squared1 ==
  I : Inport
  Q : Inport  Port=2
  AddSub1 : xbsIndex_r4/AddSub  mode=Addition  use_carryin=off  use_carryout=off  en=off  latency=addLatency  precision=User Defined  arith_type=Unsigned  n_bits=prec  bin_pt=bp  quantization=Truncate  overflow=Flag as error  dbl_ovrd=off  use_behavioral_HDL=on  pipelined=off  use_rpm=on  hw_selection=Fabric  block_type=addsub
      settings: xl_area=[9 0 0 17 0 0 0]  block_version=VER_STRING_GOES_HERE
  Mult1 : xbsIndex_r4/Mult  precision=User Defined  arith_type="Signed  (2's comp)"  n_bits=prec  bin_pt=bp+1  quantization=Truncate  overflow=Flag as error  en=off  latency=multLatency  dbl_ovrd=off  use_behavioral_HDL=off  use_embedded=on  opt=Speed  optimum_pipeline=off  pipeline=on  use_rpm=off  placement_style=Rectangular shape  block_type=mult
      settings: infoedit=Hardware notes: To use the internal pipeline stage of the dedicated multiplier you must select 'Pipeline for maximum performance'.  xl_area=[9 17 0 17 0 1 0]  block_version=VER_STRING_GOES_HERE
  Mult4 : xbsIndex_r4/Mult  precision=User Defined  arith_type="Signed  (2's comp)"  n_bits=prec  bin_pt=bp+1  quantization=Truncate  overflow=Flag as error  en=off  latency=multLatency  dbl_ovrd=off  use_behavioral_HDL=off  use_embedded=on  opt=Speed  optimum_pipeline=off  pipeline=on  use_rpm=off  placement_style=Rectangular shape  block_type=mult
      settings: infoedit=Hardware notes: To use the internal pipeline stage of the dedicated multiplier you must select 'Pipeline for maximum performance'.  xl_area=[9 17 0 17 0 1 0]  block_version=VER_STRING_GOES_HERE
  Mag : Outport
  wires:
  I/1 -> Mult4/1, Mult4/2
  Q/1 -> Mult1/1, Mult1/2
  Mult4/1 -> AddSub1/1
  Mult1/1 -> AddSub1/2
  AddSub1/1 -> Mag/1

== system ofdm_txrx_mimo_2x/OFDM Rx MIMO/Packet_Detection/Sliding Window ==
  In : Inport
  Accum1 : xbsIndex_r4/Accumulator  operation=Add  n_bits=22  overflow=Flag as error  scale=1  rst=off  hasbypass=off  en=off  dbl_ovrd=off  use_behavioral_HDL=on  implementation=Fabric  block_type=accum
      settings: infoedit="Adder or subtractor-based accumulator.   Output type and binary point position match the input.<P><P>Hardware notes: When \"Reinitialize with input 'b' on reset\" is selected, the accumulator is forced to run at the system rate even if the input 'b' is running at a slower rate."  xl_area=[12 23 0 22 0 0 0]  block_version=VER_STRING_GOES_HERE
  AddSub1 : xbsIndex_r4/AddSub  mode=Subtraction  use_carryin=off  use_carryout=off  en=off  latency=1  precision=Full  arith_type="Signed  (2's comp)"  n_bits=36  bin_pt=28  quantization=Truncate  overflow=Saturate  dbl_ovrd=off  use_behavioral_HDL=on  pipelined=off  use_rpm=off  hw_selection=Fabric  block_type=addsub
      settings: xl_area=[9 18 0 17 0 0 0]  block_version=VER_STRING_GOES_HERE
  Delay6 : xbsIndex_r4/Delay  en=off  latency=16  dbl_ovrd=off  reg_retiming=off  block_type=delay
      settings: infoedit=Hardware notes: A delay line is a chain, each link of which is an SRL16 followed by a flip-flop. If register retiming is enabled, the delay line is a chain of flip-flops.  xl_area=[9 17 0 17 0 0 0]  block_version=VER_STRING_GOES_HERE
  Out : Outport
  wires:
  Accum1/1 -> Out/1
  In/1 -> Delay6/1, AddSub1/1
  AddSub1/1 -> Accum1/1
  Delay6/1 -> AddSub1/2

== system ofdm_txrx_mimo_2x/OFDM Rx MIMO/Packet_Detection/Sliding Window1 ==
  In : Inport
  Accum1 : xbsIndex_r4/Accumulator  operation=Add  n_bits=22  overflow=Flag as error  scale=1  rst=off  hasbypass=off  en=off  dbl_ovrd=off  use_behavioral_HDL=on  implementation=Fabric  block_type=accum
      settings: infoedit="Adder or subtractor-based accumulator.   Output type and binary point position match the input.<P><P>Hardware notes: When \"Reinitialize with input 'b' on reset\" is selected, the accumulator is forced to run at the system rate even if the input 'b' is running at a slower rate."  xl_area=[12 23 0 22 0 0 0]  block_version=VER_STRING_GOES_HERE
  AddSub1 : xbsIndex_r4/AddSub  mode=Subtraction  use_carryin=off  use_carryout=off  en=off  latency=1  precision=Full  arith_type="Signed  (2's comp)"  n_bits=36  bin_pt=28  quantization=Truncate  overflow=Saturate  dbl_ovrd=off  use_behavioral_HDL=on  pipelined=off  use_rpm=off  hw_selection=Fabric  block_type=addsub
      settings: xl_area=[9 18 0 17 0 0 0]  block_version=VER_STRING_GOES_HERE
  Delay6 : xbsIndex_r4/Delay  en=off  latency=16  dbl_ovrd=off  reg_retiming=off  block_type=delay
      settings: infoedit=Hardware notes: A delay line is a chain, each link of which is an SRL16 followed by a flip-flop. If register retiming is enabled, the delay line is a chain of flip-flops.  xl_area=[9 17 0 17 0 0 0]  block_version=VER_STRING_GOES_HERE
  Out : Outport
  wires:
  Delay6/1 -> AddSub1/2
  AddSub1/1 -> Accum1/1
  In/1 -> AddSub1/1, Delay6/1
  Accum1/1 -> Out/1
